simulink library "Garbage"  (R2023a)
settings: Solver=ode45  StartTime=0.0  StopTime=10.0  RelTol=1e-3  EnableMultiTasking=on
undefined symbols (not defined in the file or in its folder): c, ending_column_index, ending_row_index, framebuffer_16x1, framebuffer_2x2, framebuffer_4x4, framebuffer_8x8, HEIGHT, hot, POS_X, POS_Y, starting_column_index, starting_row_index, WIDTH
libraries: Memory_Blocks_Lib x459, simulink x235, simulink_extras x132, dspmtrx3 x116, Bit_Multiplexers_Lib x67, Bit_Demultiplexers_Lib x49, Byte_Multiplexers_Lib x40, Miscellaneous x38, Computer_Library x17, Decoders_Lib x14, commutil2 x12, Arithmetic_Operations_Lib x8
(part 20 of 26)

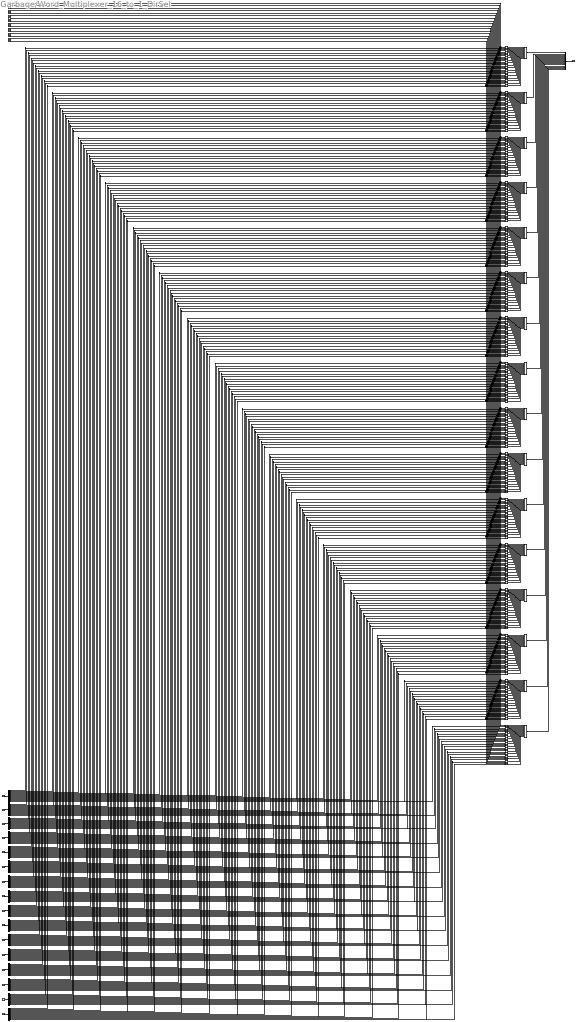
== system Garbage/Word_Multiplexer_16_to_1_DirSel ==
  note: Bit_Multiplexer_16_to_1_DirSel(Bit_15)
  note: Bit_Multiplexer_16_to_1_DirSel(Bit_14)
  note: Bit_Multiplexer_16_to_1_DirSel(Bit_13)
  note: Bit_Multiplexer_16_to_1_DirSel(Bit_12)
  note: Bit_Multiplexer_16_to_1_DirSel(Bit_11)
  note: Bit_Multiplexer_16_to_1_DirSel(Bit_10)
  note: Bit_Multiplexer_16_to_1_DirSel(Bit_09)
  note: Bit_Multiplexer_16_to_1_DirSel(Bit_08)
  note: Bit_Multiplexer_16_to_1_DirSel(Bit_07)
  note: Bit_Multiplexer_16_to_1_DirSel(Bit_06)
  note: Bit_Multiplexer_16_to_1_DirSel(Bit_05)
  note: Bit_Multiplexer_16_to_1_DirSel(Bit_04)
  note: Bit_Multiplexer_16_to_1_DirSel(Bit_03)
  note: Bit_Multiplexer_16_to_1_DirSel(Bit_02)
  note: Bit_Multiplexer_16_to_1_DirSel(Bit_01)
  note: Bit_Multiplexer_16_to_1_DirSel(Bit_00)
  Select_15(1b) : Inport
  Select_14(1b) : Inport  Port=2
  Select_13(1b) : Inport  Port=3
  Select_12(1b) : Inport  Port=4
  Select_11(1b) : Inport  Port=5
  Select_10(1b) : Inport  Port=6
  Select_09(1b) : Inport  Port=7
  Select_08(1b) : Inport  Port=8
  Select_07(1b) : Inport  Port=9
  Select_06(1b) : Inport  Port=10
  Select_05(1b) : Inport  Port=11
  Select_04(1b) : Inport  Port=12
  Select_03(1b) : Inport  Port=13
  Select_02(1b) : Inport  Port=14
  Select_01(1b) : Inport  Port=15
  Select_00(1b) : Inport  Port=16
  Data_15(2B) : Inport  Port=17
  Data_14(2B) : Inport  Port=18
  Data_13(2B) : Inport  Port=19
  Data_12(2B) : Inport  Port=20
  Data_11(2B) : Inport  Port=21
  Data_10(2B) : Inport  Port=22
  Data_09(2B) : Inport  Port=23
  Data_08(2B) : Inport  Port=24
  Data_07(2B) : Inport  Port=25
  Data_06(2B) : Inport  Port=26
  Data_05(2B) : Inport  Port=27
  Data_04(2B) : Inport  Port=28
  Data_03(2B) : Inport  Port=29
  Data_02(2B) : Inport  Port=30
  Data_01(2B) : Inport  Port=31
  Data_00(2B) : Inport  Port=32
  Bus Creator : BusCreator  Inputs=16
  Bus Selector{,1..15} (16 alike) : BusSelector  OutputSignals=signal1,signal2,signal3,signal4,signal5,signal6,signal7,signal8,signal9,signal10,signal11,signal12,signal13,signal14,signal15,signal16
  Logical Operator{1..19,21..39,41..60,62..81,83..85,87..100,102..117,119..134,136..151,153..168,170..185,187..202,204..219,221..236,238..253,255..270,272} (256 alike) : Logic  IconShape=distinctive  AllPortsSameDT=off  OutDataTypeStr=boolean
  Logical Operator{20,40,61,82,86,101,118,135,152,169,186,203,220,237,254,271} (16 alike) : Logic  Operator=OR  Inputs=16  IconShape=distinctive  OutDataTypeStr=boolean
  Data(2B) : Outport
  wires:
  Data_15(2B)/1 -> Bus Selector15/1
  Data_14(2B)/1 -> Bus Selector14/1
  Data_13(2B)/1 -> Bus Selector13/1
  Data_12(2B)/1 -> Bus Selector12/1
  Data_11(2B)/1 -> Bus Selector11/1
  Data_10(2B)/1 -> Bus Selector10/1
  Data_09(2B)/1 -> Bus Selector9/1
  Data_08(2B)/1 -> Bus Selector8/1
  Data_07(2B)/1 -> Bus Selector7/1
  Data_06(2B)/1 -> Bus Selector6/1
  Data_05(2B)/1 -> Bus Selector5/1
  Data_04(2B)/1 -> Bus Selector4/1
  Data_03(2B)/1 -> Bus Selector3/1
  Data_02(2B)/1 -> Bus Selector2/1
  Data_01(2B)/1 -> Bus Selector1/1
  Data_00(2B)/1 -> Bus Selector/1
  Bus Creator/1 -> Data(2B)/1
  Bus Selector/16 -> Logical Operator264/2  "<signal16>"
  Bus Selector1/16 -> Logical Operator263/2  "<signal16>"
  Bus Selector2/16 -> Logical Operator262/2  "<signal16>"
  Bus Selector3/16 -> Logical Operator261/2  "<signal16>"
  Bus Selector4/16 -> Logical Operator258/2  "<signal16>"
  Bus Selector5/16 -> Logical Operator257/2  "<signal16>"
  Bus Selector6/16 -> Logical Operator256/2  "<signal16>"
  Bus Selector7/16 -> Logical Operator272/2  "<signal16>"
  Bus Selector15/16 -> Logical Operator259/2  "<signal16>"
  Bus Selector14/16 -> Logical Operator260/2  "<signal16>"
  Bus Selector13/16 -> Logical Operator265/2  "<signal16>"
  Bus Selector12/16 -> Logical Operator266/2  "<signal16>"
  Bus Selector11/16 -> Logical Operator267/2  "<signal16>"
  Bus Selector10/16 -> Logical Operator268/2  "<signal16>"
  Bus Selector9/16 -> Logical Operator269/2  "<signal16>"
  Bus Selector8/16 -> Logical Operator270/2  "<signal16>"
  Bus Selector/15 -> Logical Operator247/2  "<signal15>"
  Bus Selector1/15 -> Logical Operator246/2  "<signal15>"
  Bus Selector2/15 -> Logical Operator245/2  "<signal15>"
  Bus Selector3/15 -> Logical Operator244/2  "<signal15>"
  Bus Selector4/15 -> Logical Operator241/2  "<signal15>"
  Bus Selector5/15 -> Logical Operator240/2  "<signal15>"
  Bus Selector6/15 -> Logical Operator239/2  "<signal15>"
  Bus Selector7/15 -> Logical Operator255/2  "<signal15>"
  Bus Selector15/15 -> Logical Operator242/2  "<signal15>"
  Bus Selector14/15 -> Logical Operator243/2  "<signal15>"
  Bus Selector13/15 -> Logical Operator248/2  "<signal15>"
  Bus Selector12/15 -> Logical Operator249/2  "<signal15>"
  Bus Selector11/15 -> Logical Operator250/2  "<signal15>"
  Bus Selector10/15 -> Logical Operator251/2  "<signal15>"
  Bus Selector9/15 -> Logical Operator252/2  "<signal15>"
  Bus Selector8/15 -> Logical Operator253/2  "<signal15>"
  Bus Selector/14 -> Logical Operator230/2  "<signal14>"
  Bus Selector1/14 -> Logical Operator229/2  "<signal14>"
  Bus Selector2/14 -> Logical Operator228/2  "<signal14>"
  Bus Selector3/14 -> Logical Operator227/2  "<signal14>"
  Bus Selector4/14 -> Logical Operator224/2  "<signal14>"
  Bus Selector5/14 -> Logical Operator223/2  "<signal14>"
  Bus Selector6/14 -> Logical Operator222/2  "<signal14>"
  Bus Selector7/14 -> Logical Operator238/2  "<signal14>"
  Bus Selector15/14 -> Logical Operator225/2  "<signal14>"
  Bus Selector14/14 -> Logical Operator226/2  "<signal14>"
  Bus Selector13/14 -> Logical Operator231/2  "<signal14>"
  Bus Selector12/14 -> Logical Operator232/2  "<signal14>"
  Bus Selector11/14 -> Logical Operator233/2  "<signal14>"
  Bus Selector10/14 -> Logical Operator234/2  "<signal14>"
  Bus Selector9/14 -> Logical Operator235/2  "<signal14>"
  Bus Selector8/14 -> Logical Operator236/2  "<signal14>"
  Bus Selector/13 -> Logical Operator213/2  "<signal13>"
  Bus Selector1/13 -> Logical Operator212/2  "<signal13>"
  Bus Selector2/13 -> Logical Operator211/2  "<signal13>"
  Bus Selector3/13 -> Logical Operator210/2  "<signal13>"
  Bus Selector4/13 -> Logical Operator207/2  "<signal13>"
  Bus Selector5/13 -> Logical Operator206/2  "<signal13>"
  Bus Selector6/13 -> Logical Operator205/2  "<signal13>"
  Bus Selector7/13 -> Logical Operator221/2  "<signal13>"
  Bus Selector15/13 -> Logical Operator208/2  "<signal13>"
  Bus Selector14/13 -> Logical Operator209/2  "<signal13>"
  Bus Selector13/13 -> Logical Operator214/2  "<signal13>"
  Bus Selector12/13 -> Logical Operator215/2  "<signal13>"
  Bus Selector11/13 -> Logical Operator216/2  "<signal13>"
  Bus Selector10/13 -> Logical Operator217/2  "<signal13>"
  Bus Selector9/13 -> Logical Operator218/2  "<signal13>"
  Bus Selector8/13 -> Logical Operator219/2  "<signal13>"
  Bus Selector/12 -> Logical Operator196/2  "<signal12>"
  Bus Selector1/12 -> Logical Operator195/2  "<signal12>"
  Bus Selector2/12 -> Logical Operator194/2  "<signal12>"
  Bus Selector3/12 -> Logical Operator193/2  "<signal12>"
  Bus Selector4/12 -> Logical Operator190/2  "<signal12>"
  Bus Selector5/12 -> Logical Operator189/2  "<signal12>"
  Bus Selector6/12 -> Logical Operator188/2  "<signal12>"
  Bus Selector7/12 -> Logical Operator204/2  "<signal12>"
  Bus Selector15/12 -> Logical Operator191/2  "<signal12>"
  Bus Selector14/12 -> Logical Operator192/2  "<signal12>"
  Bus Selector13/12 -> Logical Operator197/2  "<signal12>"
  Bus Selector12/12 -> Logical Operator198/2  "<signal12>"
  Bus Selector11/12 -> Logical Operator199/2  "<signal12>"
  Bus Selector10/12 -> Logical Operator200/2  "<signal12>"
  Bus Selector9/12 -> Logical Operator201/2  "<signal12>"
  Bus Selector8/12 -> Logical Operator202/2  "<signal12>"
  Bus Selector/11 -> Logical Operator179/2  "<signal11>"
  Bus Selector1/11 -> Logical Operator178/2  "<signal11>"
  Bus Selector2/11 -> Logical Operator177/2  "<signal11>"
  Bus Selector3/11 -> Logical Operator176/2  "<signal11>"
  Bus Selector4/11 -> Logical Operator173/2  "<signal11>"
  Bus Selector5/11 -> Logical Operator172/2  "<signal11>"
  Bus Selector6/11 -> Logical Operator171/2  "<signal11>"
  Bus Selector7/11 -> Logical Operator187/2  "<signal11>"
  Bus Selector15/11 -> Logical Operator174/2  "<signal11>"
  Bus Selector14/11 -> Logical Operator175/2  "<signal11>"
  Bus Selector13/11 -> Logical Operator180/2  "<signal11>"
  Bus Selector12/11 -> Logical Operator181/2  "<signal11>"
  Bus Selector11/11 -> Logical Operator182/2  "<signal11>"
  Bus Selector10/11 -> Logical Operator183/2  "<signal11>"
  Bus Selector9/11 -> Logical Operator184/2  "<signal11>"
  Bus Selector8/11 -> Logical Operator185/2  "<signal11>"
  Bus Selector/10 -> Logical Operator162/2  "<signal10>"
  Bus Selector1/10 -> Logical Operator161/2  "<signal10>"
  Bus Selector2/10 -> Logical Operator160/2  "<signal10>"
  Bus Selector3/10 -> Logical Operator159/2  "<signal10>"
  Bus Selector4/10 -> Logical Operator156/2  "<signal10>"
  Bus Selector5/10 -> Logical Operator155/2  "<signal10>"
  Bus Selector6/10 -> Logical Operator154/2  "<signal10>"
  Bus Selector7/10 -> Logical Operator170/2  "<signal10>"
  Bus Selector15/10 -> Logical Operator157/2  "<signal10>"
  Bus Selector14/10 -> Logical Operator158/2  "<signal10>"
  Bus Selector13/10 -> Logical Operator163/2  "<signal10>"
  Bus Selector12/10 -> Logical Operator164/2  "<signal10>"
  Bus Selector11/10 -> Logical Operator165/2  "<signal10>"
  Bus Selector10/10 -> Logical Operator166/2  "<signal10>"
  Bus Selector9/10 -> Logical Operator167/2  "<signal10>"
  Bus Selector8/10 -> Logical Operator168/2  "<signal10>"
  Bus Selector/9 -> Logical Operator145/2  "<signal9>"
  Bus Selector1/9 -> Logical Operator144/2  "<signal9>"
  Bus Selector2/9 -> Logical Operator143/2  "<signal9>"
  Bus Selector3/9 -> Logical Operator142/2  "<signal9>"
  Bus Selector4/9 -> Logical Operator139/2  "<signal9>"
  Bus Selector5/9 -> Logical Operator138/2  "<signal9>"
  Bus Selector6/9 -> Logical Operator137/2  "<signal9>"
  Bus Selector7/9 -> Logical Operator153/2  "<signal9>"
  Bus Selector15/9 -> Logical Operator140/2  "<signal9>"
  Bus Selector14/9 -> Logical Operator141/2  "<signal9>"
  Bus Selector13/9 -> Logical Operator146/2  "<signal9>"
  Bus Selector12/9 -> Logical Operator147/2  "<signal9>"
  Bus Selector11/9 -> Logical Operator148/2  "<signal9>"
  Bus Selector10/9 -> Logical Operator149/2  "<signal9>"
  Bus Selector9/9 -> Logical Operator150/2  "<signal9>"
  Bus Selector8/9 -> Logical Operator151/2  "<signal9>"
  Bus Selector/8 -> Logical Operator128/2  "<signal8>"
  Bus Selector1/8 -> Logical Operator127/2  "<signal8>"
  Bus Selector2/8 -> Logical Operator126/2  "<signal8>"
  Bus Selector3/8 -> Logical Operator125/2  "<signal8>"
  Bus Selector4/8 -> Logical Operator122/2  "<signal8>"
  Bus Selector5/8 -> Logical Operator121/2  "<signal8>"
  Bus Selector6/8 -> Logical Operator120/2  "<signal8>"
  Bus Selector7/8 -> Logical Operator136/2  "<signal8>"
  Bus Selector15/8 -> Logical Operator123/2  "<signal8>"
  Bus Selector14/8 -> Logical Operator124/2  "<signal8>"
  Bus Selector13/8 -> Logical Operator129/2  "<signal8>"
  Bus Selector12/8 -> Logical Operator130/2  "<signal8>"
  Bus Selector11/8 -> Logical Operator131/2  "<signal8>"
  Bus Selector10/8 -> Logical Operator132/2  "<signal8>"
  Bus Selector9/8 -> Logical Operator133/2  "<signal8>"
  Bus Selector8/8 -> Logical Operator134/2  "<signal8>"
  Bus Selector/7 -> Logical Operator111/2  "<signal7>"
  Bus Selector1/7 -> Logical Operator110/2  "<signal7>"
  Bus Selector2/7 -> Logical Operator109/2  "<signal7>"
  Bus Selector3/7 -> Logical Operator108/2  "<signal7>"
  Bus Selector4/7 -> Logical Operator105/2  "<signal7>"
  Bus Selector5/7 -> Logical Operator104/2  "<signal7>"
  Bus Selector6/7 -> Logical Operator103/2  "<signal7>"
  Bus Selector7/7 -> Logical Operator119/2  "<signal7>"
  Bus Selector15/7 -> Logical Operator106/2  "<signal7>"
  Bus Selector14/7 -> Logical Operator107/2  "<signal7>"
  Bus Selector13/7 -> Logical Operator112/2  "<signal7>"
  Bus Selector12/7 -> Logical Operator113/2  "<signal7>"
  Bus Selector11/7 -> Logical Operator114/2  "<signal7>"
  Bus Selector10/7 -> Logical Operator115/2  "<signal7>"
  Bus Selector9/7 -> Logical Operator116/2  "<signal7>"
  Bus Selector8/7 -> Logical Operator117/2  "<signal7>"
  Bus Selector/6 -> Logical Operator94/2  "<signal6>"
  Bus Selector1/6 -> Logical Operator93/2  "<signal6>"
  Bus Selector2/6 -> Logical Operator92/2  "<signal6>"
  Bus Selector3/6 -> Logical Operator91/2  "<signal6>"
  Bus Selector4/6 -> Logical Operator88/2  "<signal6>"
  Bus Selector5/6 -> Logical Operator87/2  "<signal6>"
  Bus Selector6/6 -> Logical Operator85/2  "<signal6>"
  Bus Selector7/6 -> Logical Operator102/2  "<signal6>"
  Bus Selector15/6 -> Logical Operator89/2  "<signal6>"
  Bus Selector14/6 -> Logical Operator90/2  "<signal6>"
  Bus Selector13/6 -> Logical Operator95/2  "<signal6>"
  Bus Selector12/6 -> Logical Operator96/2  "<signal6>"
  Bus Selector11/6 -> Logical Operator97/2  "<signal6>"
  Bus Selector10/6 -> Logical Operator98/2  "<signal6>"
  Bus Selector9/6 -> Logical Operator99/2  "<signal6>"
  Bus Selector8/6 -> Logical Operator100/2  "<signal6>"
  Bus Selector/5 -> Logical Operator75/2  "<signal5>"
  Bus Selector1/5 -> Logical Operator74/2  "<signal5>"
  Bus Selector2/5 -> Logical Operator73/2  "<signal5>"
  Bus Selector3/5 -> Logical Operator72/2  "<signal5>"
  Bus Selector4/5 -> Logical Operator69/2  "<signal5>"
  Bus Selector5/5 -> Logical Operator68/2  "<signal5>"
  Bus Selector6/5 -> Logical Operator64/2  "<signal5>"
  Bus Selector7/5 -> Logical Operator84/2  "<signal5>"
  Bus Selector15/5 -> Logical Operator70/2  "<signal5>"
  Bus Selector14/5 -> Logical Operator71/2  "<signal5>"
  Bus Selector13/5 -> Logical Operator76/2  "<signal5>"
  Bus Selector12/5 -> Logical Operator77/2  "<signal5>"
  Bus Selector11/5 -> Logical Operator78/2  "<signal5>"
  Bus Selector10/5 -> Logical Operator79/2  "<signal5>"
  Bus Selector9/5 -> Logical Operator80/2  "<signal5>"
  Bus Selector8/5 -> Logical Operator81/2  "<signal5>"
  Bus Selector/4 -> Logical Operator54/2  "<signal4>"
  Bus Selector1/4 -> Logical Operator53/2  "<signal4>"
  Bus Selector2/4 -> Logical Operator52/2  "<signal4>"
  Bus Selector3/4 -> Logical Operator51/2  "<signal4>"
  Bus Selector4/4 -> Logical Operator48/2  "<signal4>"
  Bus Selector5/4 -> Logical Operator47/2  "<signal4>"
  Bus Selector6/4 -> Logical Operator43/2  "<signal4>"
  Bus Selector7/4 -> Logical Operator63/2  "<signal4>"
  Bus Selector15/4 -> Logical Operator49/2  "<signal4>"
  Bus Selector14/4 -> Logical Operator50/2  "<signal4>"
  Bus Selector13/4 -> Logical Operator55/2  "<signal4>"
  Bus Selector12/4 -> Logical Operator56/2  "<signal4>"
  Bus Selector11/4 -> Logical Operator57/2  "<signal4>"
  Bus Selector10/4 -> Logical Operator58/2  "<signal4>"
  Bus Selector9/4 -> Logical Operator59/2  "<signal4>"
  Bus Selector8/4 -> Logical Operator60/2  "<signal4>"
  Bus Selector/3 -> Logical Operator33/2  "<signal3>"
  Bus Selector1/3 -> Logical Operator32/2  "<signal3>"
  Bus Selector2/3 -> Logical Operator31/2  "<signal3>"
  Bus Selector3/3 -> Logical Operator30/2  "<signal3>"
  Bus Selector4/3 -> Logical Operator27/2  "<signal3>"
  Bus Selector5/3 -> Logical Operator26/2  "<signal3>"
  Bus Selector6/3 -> Logical Operator22/2  "<signal3>"
  Bus Selector7/3 -> Logical Operator42/2  "<signal3>"
  Bus Selector15/3 -> Logical Operator28/2  "<signal3>"
  Bus Selector14/3 -> Logical Operator29/2  "<signal3>"
  Bus Selector13/3 -> Logical Operator34/2  "<signal3>"
  Bus Selector12/3 -> Logical Operator35/2  "<signal3>"
  Bus Selector11/3 -> Logical Operator36/2  "<signal3>"
  Bus Selector10/3 -> Logical Operator37/2  "<signal3>"
  Bus Selector9/3 -> Logical Operator38/2  "<signal3>"
  Bus Selector8/3 -> Logical Operator39/2  "<signal3>"
  Bus Selector/2 -> Logical Operator13/2  "<signal2>"
  Bus Selector1/2 -> Logical Operator8/2  "<signal2>"
  Bus Selector2/2 -> Logical Operator7/2  "<signal2>"
  Bus Selector3/2 -> Logical Operator6/2  "<signal2>"
  Bus Selector4/2 -> Logical Operator3/2  "<signal2>"
  Bus Selector5/2 -> Logical Operator2/2  "<signal2>"
  Bus Selector6/2 -> Logical Operator1/2  "<signal2>"
  Bus Selector7/2 -> Logical Operator21/2  "<signal2>"
  Bus Selector15/2 -> Logical Operator4/2  "<signal2>"
  Bus Selector14/2 -> Logical Operator5/2  "<signal2>"
  Bus Selector13/2 -> Logical Operator14/2  "<signal2>"
  Bus Selector12/2 -> Logical Operator15/2  "<signal2>"
  Bus Selector11/2 -> Logical Operator16/2  "<signal2>"
  Bus Selector10/2 -> Logical Operator17/2  "<signal2>"
  Bus Selector9/2 -> Logical Operator18/2  "<signal2>"
  Bus Selector8/2 -> Logical Operator19/2  "<signal2>"
  Bus Selector/1 -> Logical Operator45/2  "<signal1>"
  Bus Selector1/1 -> Logical Operator44/2  "<signal1>"
  Bus Selector2/1 -> Logical Operator41/2  "<signal1>"
  Bus Selector3/1 -> Logical Operator25/2  "<signal1>"
  Bus Selector4/1 -> Logical Operator12/2  "<signal1>"
  Bus Selector5/1 -> Logical Operator11/2  "<signal1>"
  Bus Selector6/1 -> Logical Operator10/2  "<signal1>"
  Bus Selector7/1 -> Logical Operator9/2  "<signal1>"
  Bus Selector15/1 -> Logical Operator23/2  "<signal1>"
  Bus Selector14/1 -> Logical Operator24/2  "<signal1>"
  Bus Selector13/1 -> Logical Operator46/2  "<signal1>"
  Bus Selector12/1 -> Logical Operator62/2  "<signal1>"
  Bus Selector11/1 -> Logical Operator65/2  "<signal1>"
  Bus Selector10/1 -> Logical Operator66/2  "<signal1>"
  Bus Selector9/1 -> Logical Operator67/2  "<signal1>"
  Bus Selector8/1 -> Logical Operator83/2  "<signal1>"
  Logical Operator86/1 -> Bus Creator/1
  Logical Operator62/1 -> Logical Operator86/4
  Logical Operator46/1 -> Logical Operator86/3
  Logical Operator24/1 -> Logical Operator86/2
  Logical Operator23/1 -> Logical Operator86/1
  Select_15(1b)/1 -> Logical Operator225/1, Logical Operator259/1, Logical Operator242/1, Logical Operator208/1, Logical Operator191/1, Logical Operator174/1, Logical Operator157/1, Logical Operator140/1, Logical Operator123/1, Logical Operator106/1, Logical Operator89/1, Logical Operator70/1, Logical Operator49/1, Logical Operator28/1, Logical Operator4/1, Logical Operator23/1
  Select_14(1b)/1 -> Logical Operator260/1, Logical Operator243/1, Logical Operator226/1, Logical Operator209/1, Logical Operator192/1, Logical Operator175/1, Logical Operator158/1, Logical Operator141/1, Logical Operator124/1, Logical Operator107/1, Logical Operator90/1, Logical Operator71/1, Logical Operator50/1, Logical Operator29/1, Logical Operator5/1, Logical Operator24/1
  Select_13(1b)/1 -> Logical Operator265/1, Logical Operator248/1, Logical Operator231/1, Logical Operator214/1, Logical Operator197/1, Logical Operator180/1, Logical Operator163/1, Logical Operator146/1, Logical Operator129/1, Logical Operator112/1, Logical Operator95/1, Logical Operator76/1, Logical Operator55/1, Logical Operator34/1, Logical Operator14/1, Logical Operator46/1
  Select_12(1b)/1 -> Logical Operator266/1, Logical Operator249/1, Logical Operator232/1, Logical Operator215/1, Logical Operator198/1, Logical Operator181/1, Logical Operator164/1, Logical Operator147/1, Logical Operator130/1, Logical Operator113/1, Logical Operator96/1, Logical Operator77/1, Logical Operator56/1, Logical Operator35/1, Logical Operator15/1, Logical Operator62/1
  Logical Operator83/1 -> Logical Operator86/8
  Logical Operator67/1 -> Logical Operator86/7
  Logical Operator66/1 -> Logical Operator86/6
  Logical Operator65/1 -> Logical Operator86/5
  Select_11(1b)/1 -> Logical Operator267/1, Logical Operator250/1, Logical Operator233/1, Logical Operator216/1, Logical Operator199/1, Logical Operator182/1, Logical Operator165/1, Logical Operator148/1, Logical Operator131/1, Logical Operator114/1, Logical Operator97/1, Logical Operator78/1, Logical Operator57/1, Logical Operator36/1, Logical Operator16/1, Logical Operator65/1
  Select_10(1b)/1 -> Logical Operator268/1, Logical Operator251/1, Logical Operator234/1, Logical Operator217/1, Logical Operator200/1, Logical Operator183/1, Logical Operator166/1, Logical Operator149/1, Logical Operator132/1, Logical Operator115/1, Logical Operator98/1, Logical Operator79/1, Logical Operator58/1, Logical Operator37/1, Logical Operator17/1, Logical Operator66/1
  Select_09(1b)/1 -> Logical Operator269/1, Logical Operator252/1, Logical Operator235/1, Logical Operator218/1, Logical Operator201/1, Logical Operator184/1, Logical Operator167/1, Logical Operator150/1, Logical Operator133/1, Logical Operator116/1, Logical Operator99/1, Logical Operator80/1, Logical Operator59/1, Logical Operator38/1, Logical Operator18/1, Logical Operator67/1
  Select_08(1b)/1 -> Logical Operator270/1, Logical Operator253/1, Logical Operator236/1, Logical Operator219/1, Logical Operator202/1, Logical Operator185/1, Logical Operator168/1, Logical Operator151/1, Logical Operator134/1, Logical Operator117/1, Logical Operator100/1, Logical Operator81/1, Logical Operator60/1, Logical Operator39/1, Logical Operator19/1, Logical Operator83/1
  Logical Operator12/1 -> Logical Operator86/12
  Logical Operator11/1 -> Logical Operator86/11
  Logical Operator10/1 -> Logical Operator86/10
  Logical Operator9/1 -> Logical Operator86/9
  Select_07(1b)/1 -> Logical Operator272/1, Logical Operator255/1, Logical Operator238/1, Logical Operator221/1, Logical Operator204/1, Logical Operator187/1, Logical Operator170/1, Logical Operator153/1, Logical Operator136/1, Logical Operator119/1, Logical Operator102/1, Logical Operator84/1, Logical Operator63/1, Logical Operator42/1, Logical Operator21/1, Logical Operator9/1
  Select_06(1b)/1 -> Logical Operator256/1, Logical Operator239/1, Logical Operator222/1, Logical Operator205/1, Logical Operator188/1, Logical Operator171/1, Logical Operator154/1, Logical Operator137/1, Logical Operator120/1, Logical Operator103/1, Logical Operator85/1, Logical Operator64/1, Logical Operator43/1, Logical Operator22/1, Logical Operator1/1, Logical Operator10/1
  Select_04(1b)/1 -> Logical Operator258/1, Logical Operator241/1, Logical Operator224/1, Logical Operator207/1, Logical Operator190/1, Logical Operator173/1, Logical Operator156/1, Logical Operator139/1, Logical Operator122/1, Logical Operator105/1, Logical Operator88/1, Logical Operator69/1, Logical Operator48/1, Logical Operator27/1, Logical Operator3/1, Logical Operator12/1
  Logical Operator45/1 -> Logical Operator86/16
  Logical Operator44/1 -> Logical Operator86/15
  Logical Operator41/1 -> Logical Operator86/14
  Logical Operator25/1 -> Logical Operator86/13
  Select_03(1b)/1 -> Logical Operator261/1, Logical Operator244/1, Logical Operator227/1, Logical Operator210/1, Logical Operator193/1, Logical Operator176/1, Logical Operator159/1, Logical Operator142/1, Logical Operator125/1, Logical Operator108/1, Logical Operator91/1, Logical Operator72/1, Logical Operator51/1, Logical Operator30/1, Logical Operator6/1, Logical Operator25/1
  Select_02(1b)/1 -> Logical Operator262/1, Logical Operator245/1, Logical Operator228/1, Logical Operator211/1, Logical Operator194/1, Logical Operator177/1, Logical Operator160/1, Logical Operator143/1, Logical Operator126/1, Logical Operator109/1, Logical Operator92/1, Logical Operator73/1, Logical Operator52/1, Logical Operator31/1, Logical Operator7/1, Logical Operator41/1
  Select_00(1b)/1 -> Logical Operator264/1, Logical Operator247/1, Logical Operator230/1, Logical Operator213/1, Logical Operator196/1, Logical Operator179/1, Logical Operator162/1, Logical Operator145/1, Logical Operator128/1, Logical Operator111/1, Logical Operator94/1, Logical Operator75/1, Logical Operator54/1, Logical Operator33/1, Logical Operator13/1, Logical Operator45/1
  Logical Operator20/1 -> Bus Creator/2
  Logical Operator15/1 -> Logical Operator20/4
  Logical Operator14/1 -> Logical Operator20/3
  Logical Operator5/1 -> Logical Operator20/2
  Logical Operator4/1 -> Logical Operator20/1
  Logical Operator19/1 -> Logical Operator20/8
  Logical Operator18/1 -> Logical Operator20/7
  Logical Operator17/1 -> Logical Operator20/6
  Logical Operator16/1 -> Logical Operator20/5
  Logical Operator3/1 -> Logical Operator20/12
  Logical Operator2/1 -> Logical Operator20/11
  Logical Operator1/1 -> Logical Operator20/10
  Logical Operator21/1 -> Logical Operator20/9
  Logical Operator13/1 -> Logical Operator20/16
  Logical Operator8/1 -> Logical Operator20/15
  Logical Operator7/1 -> Logical Operator20/14
  Logical Operator6/1 -> Logical Operator20/13
  Logical Operator40/1 -> Bus Creator/3
  Logical Operator35/1 -> Logical Operator40/4
  Logical Operator34/1 -> Logical Operator40/3
  Logical Operator29/1 -> Logical Operator40/2
  Logical Operator28/1 -> Logical Operator40/1
  Logical Operator39/1 -> Logical Operator40/8
  Logical Operator38/1 -> Logical Operator40/7
  Logical Operator37/1 -> Logical Operator40/6
  Logical Operator36/1 -> Logical Operator40/5
  Logical Operator27/1 -> Logical Operator40/12
  Logical Operator26/1 -> Logical Operator40/11
  Logical Operator22/1 -> Logical Operator40/10
  Logical Operator42/1 -> Logical Operator40/9
  Logical Operator33/1 -> Logical Operator40/16
  Logical Operator32/1 -> Logical Operator40/15
  Logical Operator31/1 -> Logical Operator40/14
  Logical Operator30/1 -> Logical Operator40/13
  Logical Operator61/1 -> Bus Creator/4
  Logical Operator56/1 -> Logical Operator61/4
  Logical Operator55/1 -> Logical Operator61/3
  Logical Operator50/1 -> Logical Operator61/2
  Logical Operator49/1 -> Logical Operator61/1
  Logical Operator60/1 -> Logical Operator61/8
  Logical Operator59/1 -> Logical Operator61/7
  Logical Operator58/1 -> Logical Operator61/6
  Logical Operator57/1 -> Logical Operator61/5
  Logical Operator48/1 -> Logical Operator61/12
  Logical Operator47/1 -> Logical Operator61/11
  Logical Operator43/1 -> Logical Operator61/10
  Logical Operator63/1 -> Logical Operator61/9
  Logical Operator54/1 -> Logical Operator61/16
  Logical Operator53/1 -> Logical Operator61/15
  Logical Operator52/1 -> Logical Operator61/14
  Logical Operator51/1 -> Logical Operator61/13
  Logical Operator82/1 -> Bus Creator/5
  Logical Operator77/1 -> Logical Operator82/4
  Logical Operator76/1 -> Logical Operator82/3
  Logical Operator71/1 -> Logical Operator82/2
  Logical Operator70/1 -> Logical Operator82/1
  Logical Operator81/1 -> Logical Operator82/8
  Logical Operator80/1 -> Logical Operator82/7
  Logical Operator79/1 -> Logical Operator82/6
  Logical Operator78/1 -> Logical Operator82/5
  Logical Operator69/1 -> Logical Operator82/12
  Logical Operator68/1 -> Logical Operator82/11
  Logical Operator64/1 -> Logical Operator82/10
  Logical Operator84/1 -> Logical Operator82/9
  Logical Operator75/1 -> Logical Operator82/16
  Logical Operator74/1 -> Logical Operator82/15
  Logical Operator73/1 -> Logical Operator82/14
  Logical Operator72/1 -> Logical Operator82/13
  Logical Operator101/1 -> Bus Creator/6
  Logical Operator96/1 -> Logical Operator101/4
  Logical Operator95/1 -> Logical Operator101/3
  Logical Operator90/1 -> Logical Operator101/2
  Logical Operator89/1 -> Logical Operator101/1
  Logical Operator100/1 -> Logical Operator101/8
  Logical Operator99/1 -> Logical Operator101/7
  Logical Operator98/1 -> Logical Operator101/6
  Logical Operator97/1 -> Logical Operator101/5
  Logical Operator88/1 -> Logical Operator101/12
  Logical Operator87/1 -> Logical Operator101/11
  Logical Operator85/1 -> Logical Operator101/10
  Logical Operator102/1 -> Logical Operator101/9
  Logical Operator94/1 -> Logical Operator101/16
  Logical Operator93/1 -> Logical Operator101/15
  Logical Operator92/1 -> Logical Operator101/14
  Logical Operator91/1 -> Logical Operator101/13
  Logical Operator113/1 -> Logical Operator118/4
  Logical Operator112/1 -> Logical Operator118/3
  Logical Operator107/1 -> Logical Operator118/2
  Logical Operator106/1 -> Logical Operator118/1
  Logical Operator117/1 -> Logical Operator118/8
  Logical Operator116/1 -> Logical Operator118/7
  Logical Operator115/1 -> Logical Operator118/6
  Logical Operator114/1 -> Logical Operator118/5
  Logical Operator105/1 -> Logical Operator118/12
  Logical Operator104/1 -> Logical Operator118/11
  Logical Operator103/1 -> Logical Operator118/10
  Logical Operator119/1 -> Logical Operator118/9
  Logical Operator111/1 -> Logical Operator118/16
  Logical Operator110/1 -> Logical Operator118/15
  Logical Operator109/1 -> Logical Operator118/14
  Logical Operator108/1 -> Logical Operator118/13
  Logical Operator135/1 -> Bus Creator/8
  Logical Operator130/1 -> Logical Operator135/4
  Logical Operator129/1 -> Logical Operator135/3
  Logical Operator124/1 -> Logical Operator135/2
  Logical Operator123/1 -> Logical Operator135/1
  Logical Operator134/1 -> Logical Operator135/8
  Logical Operator133/1 -> Logical Operator135/7
  Logical Operator132/1 -> Logical Operator135/6
  Logical Operator131/1 -> Logical Operator135/5
  Logical Operator122/1 -> Logical Operator135/12
  Logical Operator121/1 -> Logical Operator135/11
  Logical Operator120/1 -> Logical Operator135/10
  Logical Operator136/1 -> Logical Operator135/9
  Logical Operator128/1 -> Logical Operator135/16
  Logical Operator127/1 -> Logical Operator135/15
  Logical Operator126/1 -> Logical Operator135/14
  Logical Operator125/1 -> Logical Operator135/13
  Logical Operator147/1 -> Logical Operator152/4
  Logical Operator146/1 -> Logical Operator152/3
  Logical Operator141/1 -> Logical Operator152/2
  Logical Operator140/1 -> Logical Operator152/1
  Logical Operator151/1 -> Logical Operator152/8
  Logical Operator150/1 -> Logical Operator152/7
  Logical Operator149/1 -> Logical Operator152/6
  Logical Operator148/1 -> Logical Operator152/5
  Logical Operator139/1 -> Logical Operator152/12
  Logical Operator138/1 -> Logical Operator152/11
  Logical Operator137/1 -> Logical Operator152/10
  Logical Operator153/1 -> Logical Operator152/9
  Logical Operator145/1 -> Logical Operator152/16
  Logical Operator144/1 -> Logical Operator152/15
  Logical Operator143/1 -> Logical Operator152/14
  Logical Operator142/1 -> Logical Operator152/13
  Logical Operator169/1 -> Bus Creator/10
  Logical Operator164/1 -> Logical Operator169/4
  Logical Operator163/1 -> Logical Operator169/3
  Logical Operator158/1 -> Logical Operator169/2
  Logical Operator157/1 -> Logical Operator169/1
  Logical Operator168/1 -> Logical Operator169/8
  Logical Operator167/1 -> Logical Operator169/7
  Logical Operator166/1 -> Logical Operator169/6
  Logical Operator165/1 -> Logical Operator169/5
  Logical Operator156/1 -> Logical Operator169/12
  Logical Operator155/1 -> Logical Operator169/11
  Logical Operator154/1 -> Logical Operator169/10
  Logical Operator170/1 -> Logical Operator169/9
  Logical Operator162/1 -> Logical Operator169/16
  Logical Operator161/1 -> Logical Operator169/15
  Logical Operator160/1 -> Logical Operator169/14
  Logical Operator159/1 -> Logical Operator169/13
  Logical Operator181/1 -> Logical Operator186/4
  Logical Operator180/1 -> Logical Operator186/3
  Logical Operator175/1 -> Logical Operator186/2
  Logical Operator174/1 -> Logical Operator186/1
  Logical Operator185/1 -> Logical Operator186/8
  Logical Operator184/1 -> Logical Operator186/7
  Logical Operator183/1 -> Logical Operator186/6
  Logical Operator182/1 -> Logical Operator186/5
  Logical Operator173/1 -> Logical Operator186/12
  Logical Operator172/1 -> Logical Operator186/11
  Logical Operator171/1 -> Logical Operator186/10
  Logical Operator187/1 -> Logical Operator186/9
  Logical Operator179/1 -> Logical Operator186/16
  Logical Operator178/1 -> Logical Operator186/15
  Logical Operator177/1 -> Logical Operator186/14
  Logical Operator176/1 -> Logical Operator186/13
  Logical Operator203/1 -> Bus Creator/12
  Logical Operator198/1 -> Logical Operator203/4
  Logical Operator197/1 -> Logical Operator203/3
  Logical Operator192/1 -> Logical Operator203/2
  Logical Operator191/1 -> Logical Operator203/1
  Logical Operator202/1 -> Logical Operator203/8
  Logical Operator201/1 -> Logical Operator203/7
  Logical Operator200/1 -> Logical Operator203/6
  Logical Operator199/1 -> Logical Operator203/5
  Logical Operator190/1 -> Logical Operator203/12
  Logical Operator189/1 -> Logical Operator203/11
  Logical Operator188/1 -> Logical Operator203/10
  Logical Operator204/1 -> Logical Operator203/9
  Logical Operator196/1 -> Logical Operator203/16
  Logical Operator195/1 -> Logical Operator203/15
  Logical Operator194/1 -> Logical Operator203/14
  Logical Operator193/1 -> Logical Operator203/13
  Logical Operator215/1 -> Logical Operator220/4
  Logical Operator214/1 -> Logical Operator220/3
  Logical Operator209/1 -> Logical Operator220/2
  Logical Operator208/1 -> Logical Operator220/1
  Logical Operator219/1 -> Logical Operator220/8
  Logical Operator218/1 -> Logical Operator220/7
  Logical Operator217/1 -> Logical Operator220/6
  Logical Operator216/1 -> Logical Operator220/5
  Logical Operator207/1 -> Logical Operator220/12
  Logical Operator206/1 -> Logical Operator220/11
  Logical Operator205/1 -> Logical Operator220/10
  Logical Operator221/1 -> Logical Operator220/9
  Logical Operator213/1 -> Logical Operator220/16
  Logical Operator212/1 -> Logical Operator220/15
  Logical Operator211/1 -> Logical Operator220/14
  Logical Operator210/1 -> Logical Operator220/13
  Logical Operator237/1 -> Bus Creator/14
  Logical Operator232/1 -> Logical Operator237/4
  Logical Operator231/1 -> Logical Operator237/3
  Logical Operator226/1 -> Logical Operator237/2
  Logical Operator225/1 -> Logical Operator237/1
  Logical Operator236/1 -> Logical Operator237/8
  Logical Operator235/1 -> Logical Operator237/7
  Logical Operator234/1 -> Logical Operator237/6
  Logical Operator233/1 -> Logical Operator237/5
  Logical Operator224/1 -> Logical Operator237/12
  Logical Operator223/1 -> Logical Operator237/11
  Logical Operator222/1 -> Logical Operator237/10
  Logical Operator238/1 -> Logical Operator237/9
  Logical Operator230/1 -> Logical Operator237/16
  Logical Operator229/1 -> Logical Operator237/15
  Logical Operator228/1 -> Logical Operator237/14
  Logical Operator227/1 -> Logical Operator237/13
  Logical Operator249/1 -> Logical Operator254/4
  Logical Operator248/1 -> Logical Operator254/3
  Logical Operator243/1 -> Logical Operator254/2
  Logical Operator242/1 -> Logical Operator254/1
  Logical Operator253/1 -> Logical Operator254/8
  Logical Operator252/1 -> Logical Operator254/7
  Logical Operator251/1 -> Logical Operator254/6
  Logical Operator250/1 -> Logical Operator254/5
  Logical Operator241/1 -> Logical Operator254/12
  Logical Operator240/1 -> Logical Operator254/11
  Logical Operator239/1 -> Logical Operator254/10
  Logical Operator255/1 -> Logical Operator254/9
  Logical Operator247/1 -> Logical Operator254/16
  Logical Operator246/1 -> Logical Operator254/15
  Logical Operator245/1 -> Logical Operator254/14
  Logical Operator244/1 -> Logical Operator254/13
  Logical Operator271/1 -> Bus Creator/16
  Logical Operator266/1 -> Logical Operator271/4
  Logical Operator265/1 -> Logical Operator271/3
  Logical Operator260/1 -> Logical Operator271/2
  Logical Operator259/1 -> Logical Operator271/1
  Logical Operator270/1 -> Logical Operator271/8
  Logical Operator269/1 -> Logical Operator271/7
  Logical Operator268/1 -> Logical Operator271/6
  Logical Operator267/1 -> Logical Operator271/5
  Logical Operator258/1 -> Logical Operator271/12
  Logical Operator257/1 -> Logical Operator271/11
  Logical Operator256/1 -> Logical Operator271/10
  Logical Operator272/1 -> Logical Operator271/9
  Logical Operator264/1 -> Logical Operator271/16
  Logical Operator263/1 -> Logical Operator271/15
  Logical Operator262/1 -> Logical Operator271/14
  Logical Operator261/1 -> Logical Operator271/13
  Select_01(1b)/1 -> Logical Operator263/1, Logical Operator246/1, Logical Operator229/1, Logical Operator212/1, Logical Operator195/1, Logical Operator178/1, Logical Operator161/1, Logical Operator144/1, Logical Operator127/1, Logical Operator110/1, Logical Operator93/1, Logical Operator74/1, Logical Operator53/1, Logical Operator32/1, Logical Operator8/1, Logical Operator44/1
  Select_05(1b)/1 -> Logical Operator257/1, Logical Operator240/1, Logical Operator223/1, Logical Operator206/1, Logical Operator189/1, Logical Operator172/1, Logical Operator155/1, Logical Operator138/1, Logical Operator121/1, Logical Operator104/1, Logical Operator87/1, Logical Operator68/1, Logical Operator47/1, Logical Operator26/1, Logical Operator2/1, Logical Operator11/1
  Logical Operator254/1 -> Bus Creator/15
  Logical Operator220/1 -> Bus Creator/13
  Logical Operator186/1 -> Bus Creator/11
  Logical Operator152/1 -> Bus Creator/9
  Logical Operator118/1 -> Bus Creator/7

== system Garbage/Word_Multiplexer_2_to_1 ==
  note: Bit_Multiplexer_2_to_1(Bit_15)
  note: Bit_Multiplexer_2_to_1(Bit_14)
  note: Bit_Multiplexer_2_to_1(Bit_13)
  note: Bit_Multiplexer_2_to_1(Bit_12)
  note: Bit_Multiplexer_2_to_1(Bit_11)
  note: Bit_Multiplexer_2_to_1(Bit_10)
  note: Bit_Multiplexer_2_to_1(Bit_09)
  note: Bit_Multiplexer_2_to_1(Bit_08)
  note: Bit_Multiplexer_2_to_1(Bit_07)
  note: Bit_Multiplexer_2_to_1(Bit_06)
  note: Bit_Multiplexer_2_to_1(Bit_05)
  note: Bit_Multiplexer_2_to_1(Bit_04)
  note: Bit_Multiplexer_2_to_1(Bit_03)
  note: Bit_Multiplexer_2_to_1(Bit_02)
  note: Bit_Multiplexer_2_to_1(Bit_01)
  note: Bit_Multiplexer_2_to_1(Bit_00)
  Address(1b) : Inport
  Data_In_1(2B) : Inport  Port=2
  Data_In_0(2B) : Inport  Port=3
  Bus Creator : BusCreator  Inputs=16
  Bus Selector : BusSelector  OutputSignals=signal1,signal2,signal3,signal4,signal5,signal6,signal7,signal8,signal9,signal10,signal11,signal12,signal13,signal14,signal15,signal16
  Bus Selector1 : BusSelector  OutputSignals=signal1,signal2,signal3,signal4,signal5,signal6,signal7,signal8,signal9,signal10,signal11,signal12,signal13,signal14,signal15,signal16
  Logical Operator{2..5,9..10,14..15,18..19,22..23,26..27,30..31,34..35,38..39,42..43,46..47,50..51,54..55,58..59,62..63} (32 alike) : Logic  IconShape=distinctive  AllPortsSameDT=off  OutDataTypeStr=boolean
  Logical Operator{6,8,11,16,20,24,28,32,36,40,44,48,52,56,60,64} (16 alike) : Logic  Operator=OR  IconShape=distinctive  OutDataTypeStr=boolean
  Logical Operator61 : Logic  Operator=NOT  Inputs=4  IconShape=distinctive  AllPortsSameDT=off  OutDataTypeStr=boolean
  Data_Out(2B) : Outport
  wires:
  Logical Operator64/1 -> Bus Creator/1
  Logical Operator60/1 -> Bus Creator/2
  Logical Operator56/1 -> Bus Creator/3
  Logical Operator52/1 -> Bus Creator/4
  Logical Operator48/1 -> Bus Creator/5
  Logical Operator44/1 -> Bus Creator/6
  Logical Operator40/1 -> Bus Creator/7
  Logical Operator36/1 -> Bus Creator/8
  Data_In_0(2B)/1 -> Bus Selector1/1
  Data_In_1(2B)/1 -> Bus Selector/1
  Logical Operator8/1 -> Bus Creator/16
  Logical Operator6/1 -> Bus Creator/15
  Logical Operator11/1 -> Bus Creator/14
  Logical Operator16/1 -> Bus Creator/13
  Logical Operator32/1 -> Bus Creator/9
  Logical Operator28/1 -> Bus Creator/10
  Logical Operator24/1 -> Bus Creator/11
  Logical Operator20/1 -> Bus Creator/12
  Bus Creator/1 -> Data_Out(2B)/1
  Logical Operator61/1 -> Logical Operator3/2, Logical Operator5/2, Logical Operator10/2, Logical Operator15/2, Logical Operator19/2, Logical Operator23/2, Logical Operator27/2, Logical Operator31/2, Logical Operator35/2, Logical Operator39/2, Logical Operator43/2, Logical Operator47/2, Logical Operator51/2, Logical Operator55/2, Logical Operator59/2, Logical Operator63/2
  Bus Selector/16 -> Logical Operator2/1  "<signal16>"
  Bus Selector1/16 -> Logical Operator3/1  "<signal16>"
  Logical Operator3/1 -> Logical Operator8/2
  Logical Operator2/1 -> Logical Operator8/1
  Bus Selector/15 -> Logical Operator4/1  "<signal15>"
  Bus Selector1/15 -> Logical Operator5/1  "<signal15>"
  Logical Operator5/1 -> Logical Operator6/2
  Logical Operator4/1 -> Logical Operator6/1
  Bus Selector/14 -> Logical Operator9/1  "<signal14>"
  Bus Selector1/14 -> Logical Operator10/1  "<signal14>"
  Logical Operator10/1 -> Logical Operator11/2
  Logical Operator9/1 -> Logical Operator11/1
  Bus Selector/13 -> Logical Operator14/1  "<signal13>"
  Bus Selector1/13 -> Logical Operator15/1  "<signal13>"
  Logical Operator15/1 -> Logical Operator16/2
  Logical Operator14/1 -> Logical Operator16/1
  Bus Selector/12 -> Logical Operator18/1  "<signal12>"
  Bus Selector1/12 -> Logical Operator19/1  "<signal12>"
  Logical Operator19/1 -> Logical Operator20/2
  Logical Operator18/1 -> Logical Operator20/1
  Bus Selector/11 -> Logical Operator22/1  "<signal11>"
  Bus Selector1/11 -> Logical Operator23/1  "<signal11>"
  Logical Operator23/1 -> Logical Operator24/2
  Logical Operator22/1 -> Logical Operator24/1
  Bus Selector/10 -> Logical Operator26/1  "<signal10>"
  Bus Selector1/10 -> Logical Operator27/1  "<signal10>"
  Logical Operator27/1 -> Logical Operator28/2
  Logical Operator26/1 -> Logical Operator28/1
  Bus Selector/9 -> Logical Operator30/1  "<signal9>"
  Bus Selector1/9 -> Logical Operator31/1  "<signal9>"
  Logical Operator31/1 -> Logical Operator32/2
  Logical Operator30/1 -> Logical Operator32/1
  Bus Selector/8 -> Logical Operator34/1  "<signal8>"
  Bus Selector1/8 -> Logical Operator35/1  "<signal8>"
  Logical Operator35/1 -> Logical Operator36/2
  Logical Operator34/1 -> Logical Operator36/1
  Bus Selector/7 -> Logical Operator38/1  "<signal7>"
  Bus Selector1/7 -> Logical Operator39/1  "<signal7>"
  Logical Operator39/1 -> Logical Operator40/2
  Logical Operator38/1 -> Logical Operator40/1
  Bus Selector/6 -> Logical Operator42/1  "<signal6>"
  Bus Selector1/6 -> Logical Operator43/1  "<signal6>"
  Logical Operator43/1 -> Logical Operator44/2
  Logical Operator42/1 -> Logical Operator44/1
  Bus Selector/5 -> Logical Operator46/1  "<signal5>"
  Bus Selector1/5 -> Logical Operator47/1  "<signal5>"
  Logical Operator47/1 -> Logical Operator48/2
  Logical Operator46/1 -> Logical Operator48/1
  Bus Selector/4 -> Logical Operator50/1  "<signal4>"
  Bus Selector1/4 -> Logical Operator51/1  "<signal4>"
  Logical Operator51/1 -> Logical Operator52/2
  Logical Operator50/1 -> Logical Operator52/1
  Bus Selector/3 -> Logical Operator54/1  "<signal3>"
  Bus Selector1/3 -> Logical Operator55/1  "<signal3>"
  Logical Operator55/1 -> Logical Operator56/2
  Logical Operator54/1 -> Logical Operator56/1
  Bus Selector/2 -> Logical Operator58/1  "<signal2>"
  Bus Selector1/2 -> Logical Operator59/1  "<signal2>"
  Logical Operator59/1 -> Logical Operator60/2
  Logical Operator58/1 -> Logical Operator60/1
  Address(1b)/1 -> Logical Operator22/2, Logical Operator18/2, Logical Operator2/2, Logical Operator4/2, Logical Operator9/2, Logical Operator14/2, Logical Operator26/2, Logical Operator30/2, Logical Operator34/2, Logical Operator38/2, Logical Operator42/2, Logical Operator46/2, Logical Operator50/2, Logical Operator54/2, Logical Operator58/2, Logical Operator62/2, Logical Operator61/1
  Bus Selector/1 -> Logical Operator62/1  "<signal1>"
  Bus Selector1/1 -> Logical Operator63/1  "<signal1>"
  Logical Operator63/1 -> Logical Operator64/2
  Logical Operator62/1 -> Logical Operator64/1
